simulink library "Renault_Library"  (Simulink 9.2)
settings: Solver=ode3  StartTime=0.0  StopTime=10.0  FixedStep=0.020  RelTol=1e-3  EnableMultiTasking=on
undefined symbols (not defined in the file or in its folder): ABC, AssystemProposal, b, chkval, CoeffA_C, CoeffB_C, CoeffMean_C, ConfTime_C, DiagDelay_C, DividePercent, FailureConfActive_C, FallingEdgeDuration_C, HighLevelThresholdVoltage_C, HighLevelVoltage_C, HighLevelVoltageTunable_C, InitValue_C, InputVoltRangeMax_C, InputVoltRangeMin_C, LowActiveContact_C, LowLevelThresholdVoltage_C, LowLevelVoltage_C, MaxOperatingVoltage_C, MinOperatingVoltage_C, mV2V_conversion, OUT1, OutOfRangeHigh_C, OutOfRangeLow_C, PinSupplySaturationMax, PinSupplySaturationMin, Res1Value_C, Res2Value_C, Res3Value_C, RisingEdgeDuration_C, RSA_CANSignalFailMonitoring, SampleTime_C, service14Table, service19Table, service22Table, service24Table, service28Table, service2ETable, service2FTable, service31Table, service87Table, SizeXStateDID2FTable, SizeXTable14, SizeXTable19, SizeXTable22, SizeXTable24, SizeXTable28, SizeXTable2E, SizeXTable2F, SizeXTable31, SizeXTable87, SizeYStateDID2FTable, SizeYTable14, SizeYTable19, SizeYTable22, SizeYTable24, SizeYTable28 ... (+22)
undefined symbols (not defined in the file): BatteryVoltage_ActivationThreshold_C, FrameStatus_AbsentValue_C, FrameStatus_ConfAbsentValue_C, MemThreshold_C, NAMEOFTHEMODEL_SAMPLE_TIME_CST, NbAbs1_C, NbConfAbs1_C, NbConfPres1_C, NbPres1_C, NOMDUSIGNAL_FaultConfTime_C, NOMDUSIGNAL_FaultRehabTime_C, NOMDUSIGNAL_switchvalue_C, NOMDUSIGNAL_unavailablevalue_C, PeriodFrame1_C, ResetTime_C, WakeUpTime_C
libraries: $bdroot x112, simulink x75
(part 4 of 32)

== system Renault_Library/Services ECU/Commonly_Used_Blocks ==
  RSA_ServECU_Activation : Subsystem  Tag=[LIB]  mask(RSA_ServECU_Activation){}
      mask help:
      | Inputs:  SupplyVoltage (in mV)
      | Outputs: Enable
      |
      | Enable =1 when the SupplyVoltage inside the Voltage range defined by a minimum and a maximum in the upper mask of the component.
      |
      | Version: 01.01
      mask Help:
      | Inputs:  SupplyVoltage (in mV)
      | Outputs: Enable
      | 
      | Enable =1 when the SupplyVoltage inside the Voltage range defined by a minimum and a maximum in the upper mask of the component.
      | 
      | Version: 01.01
  RSA_ServECU_EnaOutContact : Subsystem  Tag=[LIB]  mask(RSA_ServECU_EnaOutContact){}
      mask help:
      | Inputs:  SupplyVoltage (mV) / LogicalState
      | Outputs:  PhysicalOutput / PhysicalOutput_F
      |
      | LogicalState is adapted to the logical of the component (active high or active low) with the first block,
      | then the value is filtered to prevent bouncing.
      | After, following the slope parameters, two possibilities :
      | - Generate a signal without slope if the parameters are null.
      | - Generate a signal with slope between low value et high value if the parameters are different of zero.
      | Low and high values are defined within parameters of each component.
      | For the high value, a switch is used to defined the value either from a parameter or a value equal to supply voltage.
      |
      | Version: 01.01
      mask Help:
      | Inputs:  SupplyVoltage (mV) / LogicalState
      | Outputs:  PhysicalOutput / PhysicalOutput_F
      | 
      | LogicalState is adapted to the logical of the component (active high or active low) with the first block,
      | then the value is filtered to prevent bouncing.
      | After, following the slope parameters, two possibilities :
      | - Generate a signal without slope if the parameters are null.
      | - Generate a signal with slope between low value et high value if the parameters are different of zero.
      | Low and high values are defined within parameters of each component.
      | For the high value, a switch is used to defined the value either from a parameter or a value equal to supply voltage.
      | 
      | Version: 01.01
  RSA_ServECU_EnaOutContactSat : Subsystem  Tag=[LIB]  mask(RSA_ServECU_EnaOutContactSat){}
      mask help:
      | Inputs:  SupplyVoltage (mV) / SupplyVoltage_F (mV)
      | Outputs:  PhysicalOutput /  PhysicalOutput_F
      |
      | LogicalState is adapted to the logical of the component (active high or active low) with the first block,
      | then the value is filtered to prevent bouncing.
      | After, following the slope parameters, two possibilities :
      | - Generate a signal without slope if the parameters are null.
      | - Generate a signal with slope between low value and high value if the parameters are different of zero.
      | Low and high values are defined within parameters of each component.
      | For the high value, a saturation block is used. So the high value is limited to a range [SupplyVoltage – Coeff, SupplyVoltage]. Then the value corresponds to a voltage divide bridge with resistive parameters:
      | HighLevelVoltage = SupplyVoltage x (R2+R3) / (R2+R3+R1)
      | Resistive parameters are tunable by user.
      |
      | Version: 01.01
      mask Help:
      | Inputs:  SupplyVoltage (mV) / SupplyVoltage_F (mV)
      | Outputs:  PhysicalOutput /  PhysicalOutput_F
      | 
      | LogicalState is adapted to the logical of the component (active high or active low) with the first block,
      | then the value is filtered to prevent bouncing.
      | After, following the slope parameters, two possibilities :
      | - Generate a signal without slope if the parameters are null.
      | - Generate a signal with slope between low value and high value if the parameters are different of zero.
      | Low and high values are defined within parameters of each component.
      | For the high value, a saturation block is used. So the high value is limited to a range [SupplyVoltage – Coeff, SupplyVoltage]. Then the value corresponds to a voltage divide bridge with resistive parameters:
      | HighLevelVoltage = SupplyVoltage x (R2+R3) / (R2+R3+R1)
      | Resistive parameters are tunable by user.
      | 
      | Version: 01.01
  RSA_ServECU_EnabledDiagnostic : Subsystem  Tag=[LIB]  mask(RSA_ServECU_EnabledDiagnostic){}
      mask help:
      | Inputs:  LogicalState / OutputFeedback
      | Outputs:  InternalFeedback / Diagnostic
      |
      | This block is designed to get diagnostic indication based on the signal coming from LogicalState and the voltage of the OutputFeedback (converted with a voltage divide bridge).
      | Following the LogicalState, various status are defined (with parameters) to give a nominal state or a default state. Mainly, the diagnostic array corresponds to the following figure:
      | States Value            Diag. Voltage                        Diag. States
      | 5 = State_SupVoffMax_C      V > Voffmax                          SC+
      | 4 = State_Voff_C        Voffmax > V > Voffmin                     OKOFF
      | 3 = State_SupVonMax_C /State_InfVoffMin_C Voffmin > V > Vonmax Resistive SC-
      | 2 = State_Von_C         Vonmax > V > Vonmin                         OKON
      | 1 = State_InfVonMin_C           V < Vonmin                      SC
      |
      | Threshold for Diagnostic voltage can be configured by user.
      | The LogicalState is taken into account after a tunable delay corresponding to time out on D-EIPF.
      | EnabledDiagnostic uses the block FaultConfirmation to confirm or not a failure. The confirmation delay is also tunable.
      |
      | Version: 01.01
      mask Help:
      | Inputs:  LogicalState / OutputFeedback
      | Outputs:  InternalFeedback / Diagnostic
      | 
      | This block is designed to get diagnostic indication based on the signal coming from LogicalState and the voltage of the OutputFeedback (converted with a voltage divide bridge).
      | Following the LogicalState, various status are defined (with parameters) to give a nominal state or a default state. Mainly, the diagnostic array corresponds to the following figure:
      | States Value            Diag. Voltage                        Diag. States
      | 5 = State_SupVoffMax_C      V > Voffmax                          SC+
      | 4 = State_Voff_C        Voffmax > V > Voffmin                     OKOFF
      | 3 = State_SupVonMax_C /State_InfVoffMin_C Voffmin > V > Vonmax Resistive SC-
      | 2 = State_Von_C         Vonmax > V > Vonmin                         OKON
      | 1 = State_InfVonMin_C           V < Vonmin                      SC
      | 
      | Threshold for Diagnostic voltage can be configured by user.
      | The LogicalState is taken into account after a tunable delay corresponding to time out on D-EIPF.
      | EnabledDiagnostic uses the block FaultConfirmation to confirm or not a failure. The confirmation delay is also tunable.
      | 
      | Version: 01.01
  RSA_ServECU_EnabledInputContact : Subsystem  Tag=[LIB]  mask(RSA_ServECU_EnabledInputContact){}
      mask help:
      | Inputs:  SwitchVoltage (in mV)
      | Outputs: SwitchState / SwitchState_F
      |
      | SwitchState output switches between values depending on the signal coming from SwitchVoltage.
      | The various states and threshold are given by calibrations defined in the mask of each component.
      | SwitchState_F is the filtered value. this output switches between two filter calculation, following the category of the state (failure or
      | normal):
      | 1. ConfirmedDefaultState if the current state is a failure
      | 2. Filtered_value if the current state is not a failure.
      |
      | Version: 01.01
      mask Help:
      | Inputs:  SwitchVoltage (in mV)
      | Outputs: SwitchState / SwitchState_F
      | 
      | SwitchState output switches between values depending on the signal coming from SwitchVoltage.
      | The various states and threshold are given by calibrations defined in the mask of each component.
      | SwitchState_F is the filtered value. this output switches between two filter calculation, following the category of the state (failure or
      | normal):
      | 1. ConfirmedDefaultState if the current state is a failure
      | 2. Filtered_value if the current state is not a failure.
      | 
      | Version: 01.01
  RSA_ServECU_EnabledSupply : Subsystem  Tag=[LIB]  mask(RSA_ServECU_EnabledSupply){}
      mask help:
      | Inputs:  InputVoltage
      | Outputs:  OutputVoltage / OutputVoltage_F / MeanOutputVoltage
      |
      | Voltages are in millivolt (mV)
      | OutputVoltage switches between values depending on values of InputVoltage:
      | 1. If the InputVoltage is in the range Min Max: OutputVoltage= InputVoltage x CoeffA + CoeffB
      | 2. If the InputVoltage is out of the range Min Max :
      | OutputVoltage= OutOfRangeHigh if the input is above Max
      | Or OutputVoltage= OutOfRangeLow if the input is below Min
      |
      | OutputVoltage_F output is the filtered signal of OutputVoltage.
      | MeanOutputVoltage is the mean value of the signal OutputVoltage between a number of points configured by user.
      |
      | Version: 01.01
      mask Help:
      | Inputs:  InputVoltage
      | Outputs:  OutputVoltage / OutputVoltage_F / MeanOutputVoltage
      | 
      | Voltages are in millivolt (mV)
      | OutputVoltage switches between values depending on values of InputVoltage:
      | 1. If the InputVoltage is in the range Min Max: OutputVoltage= InputVoltage x CoeffA + CoeffB
      | 2. If the InputVoltage is out of the range Min Max :
      | OutputVoltage= OutOfRangeHigh if the input is above Max
      | Or OutputVoltage= OutOfRangeLow if the input is below Min
      | 
      | OutputVoltage_F output is the filtered signal of OutputVoltage.
      | MeanOutputVoltage is the mean value of the signal OutputVoltage between a number of points configured by user.
      | 
      | Version: 01.01
  RSA_ServECU_EnabledSwitch : Subsystem  Tag=[LIB]  mask(RSA_ServECU_EnabledSwitch){}
      mask help:
      | Inputs:  SwitchVoltage (in mV)
      | Outputs: LogicalState / LogicalState_F
      |
      | LogicalState_F is the filtered value of LogicalState.
      | LogicalState is the boolean correspondant to the voltage input in the port SwitchVoltage.
      | The value are given through an hysteresis calibrated in the mask of the component.
      |
      | Version: 01.01
      mask Help:
      | Inputs:  SwitchVoltage (in mV)
      | Outputs: LogicalState / LogicalState_F
      | 
      | LogicalState_F is the filtered value of LogicalState.
      | LogicalState is the boolean correspondant to the voltage input in the port SwitchVoltage.
      | The value are given through an hysteresis calibrated in the mask of the component.
      | 
      | Version: 01.01
  RSA_ServECU_FaultConfirmation : Subsystem  Tag=[LIB]  mask(RSA_ServECU_FaultConfirmation){}
      mask help:
      | Inputs:     LastConfirmedState
      |             CurrentFaultState
      |             Failure
      | Outputs:    ConfirmedFaultState
      |
      | ConfirmedFaultState recopy CurrentFaultState if the confirmation time is elapsed.
      | Otherwise it stay on previous state (LastConfirmedState).
      | Failure shall be set to "1" when a fault occured or with constant "1" if the fault confirmation filter is permanent (no use of SoftwareFiltering in the same level).
      |
      | Version: 01.01
      mask Help:
      | Inputs:     LastConfirmedState
      |             CurrentFaultState
      |             Failure
      | Outputs:    ConfirmedFaultState
      | 
      | ConfirmedFaultState recopy CurrentFaultState if the confirmation time is elapsed.
      | Otherwise it stay on previous state (LastConfirmedState).
      | Failure shall be set to "1" when a fault occured or with constant "1" if the fault confirmation filter is permanent (no use of SoftwareFiltering in the same level).
      | 
      | Version: 01.01
  RSA_ServECU_SmartFET : Subsystem  Tag=[LIB]  mask(RSA_ServECU_SmartFET){}
      mask help:
      | Inputs:
      | PwmRatio (duty cycle in percent)
      | PinSupply (mV)
      | NoSmartProtection (=1 when NoSmartProtection)
      | Outputs:
      | PhysicalOutput (mV) --> Voltage related with PwmRatio and PinSupply
      | OutputCmd (~PwmRatio) for feedback in applicative level
      |
      | Version: 01.00
      mask Help:
      | Inputs:
      | PwmRatio (duty cycle in percent)
      | PinSupply (mV)
      | NoSmartProtection (=1 when NoSmartProtection)
      | Outputs:
      | PhysicalOutput (mV) --> Voltage related with PwmRatio and PinSupply
      | OutputCmd (~PwmRatio) for feedback in applicative level
      | 
      | Version: 01.00
  RSA_ServECU_SoftwareFiltering : Subsystem  Tag=[LIB]  mask(RSA_ServECU_SoftwareFiltering){}
      mask help:
      | Inputs: filtered_value
      | Outputs: raw_value
      |
      | filtered_value is the confirmed value taking into acount the stabilization time parameter defined in the component parameters.
      | A variation of raw_value during a time shorter than stabilization time is not taken into account.
      |
      | Version: 01.01
      mask Help:
      | Inputs: filtered_value
      | Outputs: raw_value
      | 
      | filtered_value is the confirmed value taking into acount the stabilization time parameter defined in the component parameters.
      | A variation of raw_value during a time shorter than stabilization time is not taken into account.
      | 
      | Version: 01.01
